simulink library "ee_lib_for_power_systems"  (R2025b) (continued)
== system ee_lib_for_power_systems/Network Components == (continued)
      | hC = find_system(gcbh,'FollowLinks','on','FindAll','on','IncludeCommented','on','LookUnderMasks','on','Name','Capacitor');
      | 
      | set(hR,'Commented','off')
      | set(hL,'Commented','off')
      | set(hC,'Commented','off')
      | 
      | if R == Inf
      |     set(hR,'Commented','on')
      | end
      | 
      | if L == Inf
      |     set(hL,'Commented','on')
      | end
      | 
      | if C == 0
      |     set(hC,'Commented','on')
      | end
      mask Callback run_time:
      | run_time = get_param(gcbh,'run_time');
      | 
      | hR = find_system(gcbh,'FollowLinks','on','FindAll','on','IncludeCommented','on','LookUnderMasks','on','Name','Resistor');
      | hL = find_system(gcbh,'FollowLinks','on','FindAll','on','IncludeCommented','on','LookUnderMasks','on','Name','Inductor');
      | hC = find_system(gcbh,'FollowLinks','on','FindAll','on','IncludeCommented','on','LookUnderMasks','on','Name','Capacitor');
      | 
      | switch run_time
      |     case 'off'
      |         pcr = 'compiletime';
      |     case 'on'
      |         pcr = 'runtime';
      | end
      | 
      | set(hR,'R_conf',pcr)
      | set(hL,'l_conf',pcr)
      | set(hC,'c_conf',pcr)
      mask DialogControl DescGroupVar Prompt: Parallel Z - RLC
      mask DialogControl DescTextVar Prompt: Define constant parallel impedance through R, L and C values. Set R = Inf to remove the resistor, set L = Inf to remove the inductor and set C = 0 to remove the capacitor.
      mask Display: disp('Parallel Z - RLC')
  Passive : ee_lib/Passive  Tag=simscape_sublibrary  SystemSampleTime=-1  VariantActivationTime=update diagram  EmptyChoice=off  PassThrough=off  PassThroughVariantControl=(default)  EmptyChoiceVariantControl=(default)  AllowFlexibleInterface=on  Latency=0  AutoFrameSizeCalculation=off
  Pi-Section Pos-Zero (PU) : Subsystem  mask{length=1, Pnom_Vnom_Fnom=[5e6 5e3 60], R1_L1_C1=[2 0.05 1e-8], R0_L0_C0=[4 0.1 1e-09], run_time=off}
      mask prompts: length "Line Length (km)"; Pnom_Vnom_Fnom "Nominal values [Pnom(VA) Vnom(Vrms) Fnom(Hz)]"; R1_L1_C1 "Positive Sequence [R1(pu) L1(pu) C1(pu)]"; R0_L0_C0 "Zero Sequence [R0(pu) L0(pu) C0(pu)]"; run_time "Set parameters to run-time (model does not recompile if parameters change value)"
      mask init:
      | maskObj = Simulink.Mask.get(gcbh);
      | workspaceObj = maskObj.getWorkspace();
      | 
      | Pnom_Vnom_Fnom = workspaceObj.get('Pnom_Vnom_Fnom');
      | R1_L1_C1 = workspaceObj.get('R1_L1_C1');
      | R0_L0_C0 = workspaceObj.get('R0_L0_C0');
      | 
      | Pnom = Pnom_Vnom_Fnom(1);
      | Vnom = Pnom_Vnom_Fnom(2);
      | Fnom = Pnom_Vnom_Fnom(3);
      | 
      | Rbase = Vnom^2/Pnom(1);
      | Lbase = Rbase/(2*pi*Fnom);
      | Cbase = 1/Rbase/(2*pi*Fnom);
      | 
      | R1_si = R1_L1_C1(1)*Rbase;
      | L1_si = R1_L1_C1(2)*Lbase;
      | C1_si = R1_L1_C1(3)*Cbase;
      | R0_si = R0_L0_C0(1)*Rbase;
      | L0_si = R0_L0_C0(2)*Lbase;
      | C0_si = R0_L0_C0(3)*Cbase;
      | 
      | Rs_si = (2*R1_si + R0_si)/3;
      | Rm_si = (R0_si - R1_si)/3;
      | 
      | Ls_si = (2*L1_si + L0_si)/3;
      | Lm_si = (L0_si - L1_si)/3;
      | 
      | Cl_si = (C1_si-C0_si)/3;
      | Cg_si = C0_si;
      mask Callback run_time:
      | run_time = get_param(gcbh,'run_time');
      | 
      | path_line = [gcb,'/line'];
      | 
      | switch run_time
      |     case 'off'
      |         pcr = 'compiletime';
      |     case 'on'
      |         pcr = 'runtime';
      | end
      | 
      | set_param(path_line,'freq_conf',pcr)
      | set_param(path_line,'length_conf',pcr)
      | set_param(path_line,'R_conf',pcr)
      | set_param(path_line,'L_conf',pcr)
      | set_param(path_line,'Rm_conf',pcr)
      | set_param(path_line,'M_conf',pcr)
      | set_param(path_line,'Cg_conf',pcr)
      | set_param(path_line,'Cl_conf',pcr)
      mask DialogControl DescGroupVar Prompt: Pi-Section Pos-Zero
      mask DialogControl DescTextVar Prompt: Define pi-section line with positive and zero sequence values
      mask DialogControl Container5 Prompt: Parameters
      mask Display: disp('Pi-Section\nPos-Zero\n(PU)')
  Pi-Section Pos-Zero (SI) : Subsystem  mask{length=1, Fnom=60, R1_L1_C1=[0.02 0.05 1e-8], R0_L0_C0=[0.04 4 0.1 1e-09], run_time=off}
      mask prompts: length "Line Length (km)"; Fnom "Nominal Frequency (Hz)"; R1_L1_C1 "Positive Sequence [R1(Ohm/km) L1(H/km) C1(F/km)]"; R0_L0_C0 "Zero Sequence [R0(Ohm/km) L0(H/km) C0(F/km)]"; run_time "Set parameters to run-time (model does not recompile if parameters change value)"
      mask init:
      | maskObj = Simulink.Mask.get(gcbh);
      | workspaceObj = maskObj.getWorkspace();
      | 
      | R1_L1_C1 = workspaceObj.get('R1_L1_C1');
      | R0_L0_C0 = workspaceObj.get('R0_L0_C0');
      | 
      | R1_si = R1_L1_C1(1);
      | L1_si = R1_L1_C1(2);
      | C1_si = R1_L1_C1(3);
      | R0_si = R0_L0_C0(1);
      | L0_si = R0_L0_C0(2);
      | C0_si = R0_L0_C0(3);
      | 
      | Rs_si = (2*R1_si + R0_si)/3;
      | Rm_si = (R0_si - R1_si)/3;
      | 
      | Ls_si = (2*L1_si + L0_si)/3;
      | Lm_si = (L0_si - L1_si)/3;
      | 
      | Cl_si = (C1_si-C0_si)/3;
      | Cg_si = C0_si;
      mask Callback run_time:
      | run_time = get_param(gcb,'run_time');
      | 
      | path_line = [gcb,'/line'];
      | 
      | switch run_time
      |     case 'off'
      |         pcr = 'compiletime';
      |     case 'on'
      |         pcr = 'runtime';
      | end
      | 
      | set_param(path_line,'freq_conf',pcr)
      | set_param(path_line,'length_conf',pcr)
      | set_param(path_line,'R_conf',pcr)
      | set_param(path_line,'L_conf',pcr)
      | set_param(path_line,'Rm_conf',pcr)
      | set_param(path_line,'M_conf',pcr)
      | set_param(path_line,'Cg_conf',pcr)
      | set_param(path_line,'Cl_conf',pcr)
      mask DialogControl DescGroupVar Prompt: Pi-Section Pos-Zero (SI)
      mask DialogControl DescTextVar Prompt: Define pi-section line with positive and zero sequence values
      mask DialogControl Container5 Prompt: Parameters
      mask Display: disp('Pi-Section\nPos-Zero\n(SI)')
  Resistor (Table-Based) : Subsystem  mask{i_vec=[1 2 3 4], v_vec=[1 2 3 4], run_time=off}
      mask prompts: i_vec "Current vector (A)"; v_vec "Voltage vector (V)"; run_time "Set parameters to run-time (model does not recompile if parameters change value)"
      mask Callback run_time:
      | h = find_system(gcbh,'FollowLinks','on','FindAll','on','IncludeCommented','on','LookUnderMasks','on','Name','LUT');
      | 
      | run_time = get(gcbh,'run_time');
      | 
      | switch run_time
      | 
      |     case 'on'
      | 
      |         set(h,'x_conf','runtime')
      |         set(h,'f_conf','runtime')
      | 
      |     case 'off'
      | 
      |         set(h,'x_conf','compiletime')
      |         set(h,'f_conf','compiletime')
      | 
      | end
      mask Display: disp('Resistor\n(Table-Based)')
  Series Z - PQ : Subsystem  mask{Vnom_Fnom=[100 60], P=1e3, Q=1e3, run_time=off}
      mask prompts: Vnom_Fnom "Nominal Values [Vnom(Vrms) Fnom(Hz)]"; P "Active Power (W)"; Q "Reactive Power  (inductive +VAr, capacitive -VAr )"; run_time "Set parameters to run-time (model does not recompile if parameters change value)"
      mask init:
      | maskObj = Simulink.Mask.get(gcbh);
      | workspaceObj = maskObj.getWorkspace();
      | 
      | Vnom_Fnom = workspaceObj.get('Vnom_Fnom');
      | P = workspaceObj.get('P');
      | Q = workspaceObj.get('Q');
      | 
      | path_R   = [gcb,'/Resistor'];
      | path_L   = [gcb,'/Inductor'];
      | path_C   = [gcb,'/Capacitor'];
      | 
      | Vnom = Vnom_Fnom(1);
      | Fnom = Vnom_Fnom(2);
      | 
      | set_param(path_R,'Commented','off')
      | set_param(path_L,'Commented','off')
      | set_param(path_C,'Commented','off')
      | 
      | S = sqrt(P^2 + Q^2);
      | I = S/Vnom;
      | R = P/(I^2);
      | C = 1e-6;
      | L = 1e-6;
      | 
      | if P == 0
      |     set_param(path_R,'Commented','through')
      | end
      | 
      | if Q > 0
      |     L = Q/(I^2)/(2*pi*Fnom);
      |     set_param(path_C,'Commented','through')
      | elseif Q < 0
      |     C = (I^2)/abs(Q)/(2*pi*Fnom);
      |     set_param(path_L,'Commented','through')
      | elseif Q == 0
      |     set_param(path_L,'Commented','through')
      |     set_param(path_C,'Commented','through')
      | end
      mask Callback run_time:
      | run_time = get_param(gcb,'run_time');
      | 
      | path_R   = [gcb,'/Resistor'];
      | path_L   = [gcb,'/Inductor'];
      | path_C   = [gcb,'/Capacitor'];
      | 
      | switch run_time
      |     case 'off'
      |         pcr = 'compiletime';
      |     case 'on'
      |         pcr = 'runtime';
      | end
      | 
      | set_param(path_R,'R_conf',pcr)
      | set_param(path_L,'l_conf',pcr)
      | set_param(path_C,'c_conf',pcr)
      mask DialogControl DescGroupVar Prompt: Series Z - PQ
      mask DialogControl DescTextVar Prompt: Define series constant impedance through P and Q values
      mask Display: disp('Series Z - PQ')
  Series Z - RLC : Subsystem  mask{R=0, L=0.001, C=1e-5, run_time=off}
      mask prompts: R "Resistance (Ohm)"; L "Inductance (H)"; C "Capacitance (F)"; run_time "Set parameters to run-time (model does not recompile if parameters change value)"
      mask init:
      | maskObj = Simulink.Mask.get(gcbh);
      | workspaceObj = maskObj.getWorkspace();
      | 
      | R = workspaceObj.get('R');
      | L = workspaceObj.get('L');
      | C = workspaceObj.get('C');
      | 
      | 
      | hR = find_system(gcbh,'FollowLinks','on','FindAll','on','IncludeCommented','on','LookUnderMasks','on','Name','Resistor');
      | hL = find_system(gcbh,'FollowLinks','on','FindAll','on','IncludeCommented','on','LookUnderMasks','on','Name','Inductor');
      | hC = find_system(gcbh,'FollowLinks','on','FindAll','on','IncludeCommented','on','LookUnderMasks','on','Name','Capacitor');
      | 
      | set(hR,'Commented','off')
      | set(hL,'Commented','off')
      | set(hC,'Commented','off')
      | 
      | if R == 0
      |     set(hR,'Commented','through')
      | end
      | 
      | if L == 0
      |     set(hL,'Commented','through')
      | end
      | 
      | if C == Inf
      |     set(hC,'Commented','through')
      | end
      mask Callback run_time:
      | run_time = get_param(gcbh,'run_time');
      | 
      | hR = find_system(gcbh,'FollowLinks','on','FindAll','on','IncludeCommented','on','LookUnderMasks','on','Name','Resistor');
      | hL = find_system(gcbh,'FollowLinks','on','FindAll','on','IncludeCommented','on','LookUnderMasks','on','Name','Inductor');
      | hC = find_system(gcbh,'FollowLinks','on','FindAll','on','IncludeCommented','on','LookUnderMasks','on','Name','Capacitor');
      | 
      | switch run_time
      |     case 'off'
      |         pcr = 'compiletime';
      |     case 'on'
      |         pcr = 'runtime';
      | end
      | 
      | set(hR,'R_conf',pcr)
      | set(hL,'l_conf',pcr)
      | set(hC,'c_conf',pcr)
      mask DialogControl DescGroupVar Prompt: Series Z - RLC
      mask DialogControl DescTextVar Prompt: Define constant series impedance through R, L and C values. Set R = 0 to remove the resistor, set L = 0 to remove the inductor and set C = Inf to remove the capacitor.
      mask Display: disp('Series Z - RLC')
  Simulink Logic Breaker : Subsystem  mask{initB=1, Ron=1e-6, Goff=1e-6, Ts=5e-5}
      mask prompts: initB "Initial Breaker Status (0 open, 1 closed)"; Ron "On Resistance (Ohm)"; Goff "Off Conductance (1/Ohm)"; Ts "Sample Time (sec)"
      settings: PortSchema="{\"entries\":[{\"content\":{\"sides\":[{\"content\":{\"connectorIds\":[\"LConn1\"]},\"type\":\"ConnectorPlacement.EquallySpacedRectSide\",\"uuid\":\"ba0bfa42-8952-44f0-a781-b329520855ba\"},{\"content\":{\"connectorIds\":[\"RConn1\"],\"side\":\"RIGHT\"},\"type\":\"ConnectorPlacement.EquallySpacedRectSide\",\"uuid\":\"e904d15c-eff7-47ac-9c24-60f529d3b54e\"},{\"content\":{\"connectorIds\":[\"In1\"],\"side\":\"TOP\"},\"type\":\"ConnectorPlacement.EquallySpacedRectSide\",\"uuid\":\"ccdae53b-1241-4ab5-a4dd-bd861eec6f9e\"}]},\"type\":\"ConnectorPlacement.EquallySpacedConnector\",\"uuid\":\"4cc3036f-f6ff-4340-9c1c-9c60c081c1a2\"}],\"packageUris\":[\"http://schema.mathworks.com/mf0/Connector/R2020b\"],\"version\":\"1.0\"}"
      mask DialogControl DescGroupVar Prompt: Single-Phase Breaker
      mask DialogControl DescTextVar Prompt: Breaker is closed when signal is 1. Breaker opens when signal goes to zero and current crosses zero.
      mask Display: disp('Breaker')
  Simulink Logic Breaker (Three-Phase) : Subsystem  mask{initB=1, Ron=1e-6, Goff=1e-6, Ts=5e-5}
      mask prompts: initB "Initial Breaker Status (0 open, 1 closed)"; Ron "On Resistance (Ohm)"; Goff "Off Conductance (1/Ohm)"; Ts "Sample Time (sec)"
      settings: PortSchema="{\"entries\":[{\"content\":{\"sides\":[{\"content\":{\"connectorIds\":[\"LConn1\"]},\"type\":\"ConnectorPlacement.EquallySpacedRectSide\",\"uuid\":\"ba0bfa42-8952-44f0-a781-b329520855ba\"},{\"content\":{\"connectorIds\":[\"RConn1\"],\"side\":\"RIGHT\"},\"type\":\"ConnectorPlacement.EquallySpacedRectSide\",\"uuid\":\"e904d15c-eff7-47ac-9c24-60f529d3b54e\"},{\"content\":{\"connectorIds\":[\"In1\"],\"side\":\"TOP\"},\"type\":\"ConnectorPlacement.EquallySpacedRectSide\",\"uuid\":\"ccdae53b-1241-4ab5-a4dd-bd861eec6f9e\"}]},\"type\":\"ConnectorPlacement.EquallySpacedConnector\",\"uuid\":\"4cc3036f-f6ff-4340-9c1c-9c60c081c1a2\"}],\"packageUris\":[\"http://schema.mathworks.com/mf0/Connector/R2020b\"],\"version\":\"1.0\"}"
      mask DialogControl DescGroupVar Prompt: Three-Phase Breaker
      mask DialogControl DescTextVar Prompt: Breaker is closed when signal is 1. Breaker opens when signal goes to zero and current crosses zero.
      mask Display: disp('Breaker')
  Switches & Breakers : ee_lib/Switches & Breakers  Tag=simscape_sublibrary  SystemSampleTime=-1  VariantActivationTime=update diagram  EmptyChoice=off  PassThrough=off  PassThroughVariantControl=(default)  EmptyChoiceVariantControl=(default)  AllowFlexibleInterface=on  Latency=0  AutoFrameSizeCalculation=off
  Transformers : ee_lib/Passive/Transformers  Tag=simscape_sublibrary  SystemSampleTime=-1  VariantActivationTime=update diagram  EmptyChoice=off  PassThrough=off  PassThroughVariantControl=(default)  EmptyChoiceVariantControl=(default)  AllowFlexibleInterface=on  Latency=0  AutoFrameSizeCalculation=off
  Two-Winding Transformer Nameplate (Three-Phase) : Subsystem  mask{conn1=Y, conn2=Y, core=threelimb, Prated=100, Frated=60, HV_LV=[11000 415], HVA_LVA=[5.25 139.1], Zperc=3.88, load_loss=1750, no_load_loss=145, run_time=off}
      mask prompts: conn1 "Winding 1 Connection"; conn2 "Winding 2 Connection"; Prated "Rated Power (kVA)"; Frated "Rated Frequency (Hz)"; HV_LV "Rated Voltage (Vrms line-line) [HV LV]"; HVA_LVA "Rated Current (A line peak) [HV Amps LV Amps]"; Zperc "Short-Circuit Impedance (%)"; load_loss "Copper (Load) Loss (W)"; no_load_loss "Core (No-Load) Loss (W)"; run_time "Set parameters to run-time (model does not recompile if parameters change value)"
      mask init:
      | maskObj = Simulink.Mask.get(gcbh);
      | workspaceObj = maskObj.getWorkspace();
      | 
      | Prated = workspaceObj.get('Prated');
      | Frated = workspaceObj.get('Frated');
      | HV_LV = workspaceObj.get('HV_LV');
      | HVA_LVA = workspaceObj.get('HVA_LVA');
      | Zperc = workspaceObj.get('Zperc');
      | load_loss = workspaceObj.get('load_loss');
      | no_load_loss = workspaceObj.get('no_load_loss');
      | 
      | HV = HV_LV(1);
      | LV = HV_LV(2);
      | HVA = HVA_LVA(1);
      | LVA = HVA_LVA(2);
      | 
      | P_rated_peak_per_phase = sqrt(2)*Prated/3*1000;
      | V_rated_peak_phase_to_ground = HV*sqrt(2/3);
      | 
      | Rbase1_per_phase = V_rated_peak_phase_to_ground^2/P_rated_peak_per_phase;
      | 
      | Rm = V_rated_peak_phase_to_ground^2/(sqrt(2)*no_load_loss/3);
      | 
      | Rm_pu = Rm/Rbase1_per_phase;
      | 
      | R1_pu = (sqrt(2)*load_loss/3)/(HVA^2)/Rbase1_per_phase/2;
      | R2_pu = R1_pu;
      | 
      | L1_pu = sqrt((sqrt(1/2)*Zperc/100)^2-R1_pu^2);
      | L2_pu = L1_pu;
      | 
      | path_Transformer = [gcb,'/Transformer'];
      | 
      | conn1 = get_param(gcb,'conn1');
      | conn2 = get_param(gcb,'conn2');
      | core  = get_param(gcb,'core');
      | 
      | set_param(path_Transformer,'Winding1Connection',['ee.enum.windingconnection.',conn1])
      | set_param(path_Transformer,'Winding2Connection',['ee.enum.windingconnection.',conn2])
      | set_param(path_Transformer,'CoreType',['ee.enum.coretype.',core])
      mask TypeOptions conn1: Y | Yg | delta1 | delta11
      mask TypeOptions conn2: Y | Yg | delta1 | delta11
      mask TypeOptions core: threelimb | fivelimb
      mask DialogControl DescGroupVar Prompt: Two-Winding Transformer (Nameplate)
      mask DialogControl Container4 Prompt: Configure
      mask DialogControl Container5 Prompt: Nameplate Data
      mask Display:
      | color('lightblue')
      | 
      | ph = linspace(0,2*pi,200);
      | 
      | plot(0.15*sin(ph)+0.4,0.15*cos(ph)+0.5)
      | plot(0.15*sin(ph)+0.6,0.15*cos(ph)+0.5)
      | plot([0.2 0.25],[0.5 0.5])
      | plot([0.75 0.8],[0.5 0.5])
      | plot(0,0)
      | plot(1,1)
      | 
      | conn1 = get_param(gcbh,'conn1');
      | cc1 = conn1;
      | if strcmp(conn1,'delta1')
      |     cc1 = 'D1';
      | elseif strcmp(conn1,'delta11')
      |     cc1 = 'D11';
      | end
      | 
      | text(0.2,0.25,cc1)
      | 
      | conn2 = get_param(gcbh,'conn2');
      | cc2 = conn2;
      | if strcmp(conn2,'delta1')
      |     cc2 = 'D1';
      | elseif strcmp(conn2,'delta11')
      |     cc2 = 'D11';
      | end
      | 
      | text(0.7,0.25,cc2)
      | 
      | core = get_param(gcbh,'core');
      | if strcmp(core,'threelimb')
      |     co1 = '3-Limb';
      | elseif strcmp(core,'fivelimb')
      |     co1 = '5-Limb';
      | end
      | 
      | text(0.32,0.8,co1)
  Two-Winding Transformer PU (Three-Phase) : Subsystem  mask{conn1=Yg, conn2=delta1, core=threelimb, Pnom_Fnom=[250e6 60], V1_V2=[500000 250000], R_X=[0.01 0.05], Rm_Xm=[500 500], run_time=off}
      mask prompts: conn1 "Winding 1 Connection"; conn2 "Winding 2 Connection"; Pnom_Fnom "Nominal values [Pnom(VA) Fnom(Hz)]"; V1_V2 "Primary and Secondary Voltages[V1(Vrms) V2(Vrms)]"; R_X "Winding Resistance and Leakage Reactance [R(pu) X(pu)]"; Rm_Xm "Magnetization [Rm(pu) Xm(pu)]"; run_time "Set parameters to run-time (model does not recompile if parameters change value)"
      mask init:
      | maskObj = Simulink.Mask.get(gcbh);
      | workspaceObj = maskObj.getWorkspace();
      | 
      | Pnom_Fnom = workspaceObj.get('Pnom_Fnom');
      | V1_V2 = workspaceObj.get('V1_V2');
      | R_X = workspaceObj.get('R_X');
      | Rm_Xm = workspaceObj.get('Rm_Xm');
      | 
      | V1 = V1_V2(1);
      | V2 = V1_V2(2);
      | 
      | R1_pu = R_X(1)/2;
      | L1_pu = R_X(2)/2;
      | R2_pu = R_X(1)/2;
      | L2_pu = R_X(2)/2;
      | Rm_pu = Rm_Xm(1);
      | Lm_pu = Rm_Xm(2);
      | 
      | conn1 = get_param(gcb,'conn1');
      | conn2 = get_param(gcb,'conn2');
      | core  = get_param(gcb,'core');
      | 
      | 
      | path_Transformer = [gcb,'/Transformer'];
      | 
      | set_param(path_Transformer,'Winding1Connection',['ee.enum.windingconnection.',conn1])
      | set_param(path_Transformer,'Winding2Connection',['ee.enum.windingconnection.',conn2])
      | set_param(path_Transformer,'CoreType',['ee.enum.coretype.',core])
      mask TypeOptions conn1: Y | Yg | delta1 | delta11
      mask TypeOptions conn2: Y | Yg | delta1 | delta11
      mask TypeOptions core: threelimb | fivelimb
      mask Callback run_time:
      | run_time = get_param(gcb,'run_time');
      | 
      | path_Transformer = [gcb,'/Transformer'];
      | 
      | switch run_time
      |     case 'off'
      |         pcr = 'compiletime';
      |     case 'on'
      |         pcr = 'runtime';
      | end
      | 
      | set_param(path_Transformer,'VRated1_conf',pcr)
      | set_param(path_Transformer,'VRated2_conf',pcr)
      | set_param(path_Transformer,'SRated_conf',pcr)
      | set_param(path_Transformer,'FRated_conf',pcr)
      | set_param(path_Transformer,'pu_Rm_conf',pcr)
      | set_param(path_Transformer,'pu_Xm_conf',pcr)
      | set_param(path_Transformer,'pu_Rw1_conf',pcr)
      | set_param(path_Transformer,'pu_Xl1_conf',pcr)
      | set_param(path_Transformer,'pu_Rw2_conf',pcr)
      | set_param(path_Transformer,'pu_Xl2_conf',pcr)
      mask DialogControl DescGroupVar Prompt: Two-Winding Transformer
      mask DialogControl DescTextVar Prompt: Winding resistance and leakage reactance are split equally between primary and secondary windings.
      mask DialogControl Container4 Prompt: Configure
      mask DialogControl Container5 Prompt: Parameters
      mask Display:
      | color('lightblue')
      | 
      | ph = linspace(0,2*pi,200);
      | 
      | plot(0.2*sin(ph)+0.4,0.2*cos(ph)+0.5)
      | plot(0.2*sin(ph)+0.6,0.2*cos(ph)+0.5)
      | plot([0.1 0.2],[0.5 0.5])
      | plot([0.8 0.9],[0.5 0.5])
      | plot(0,0)
      | plot(1,1)
      | 
      | conn1 = get_param(gcbh,'conn1');
      | cc1 = conn1;
      | if strcmp(conn1,'delta1')
      |     cc1 = 'D1';
      | elseif strcmp(conn1,'delta11')
      |     cc1 = 'D11';
      | end
      | 
      | text(0.2,0.25,cc1)
      | 
      | conn2 = get_param(gcbh,'conn2');
      | cc2 = conn2;
      | if strcmp(conn2,'delta1')
      |     cc2 = 'D1';
      | elseif strcmp(conn2,'delta11')
      |     cc2 = 'D11';
      | end
      | 
      | text(0.7,0.25,cc2)
      | 
      | core = get_param(gcbh,'core');
      | if strcmp(core,'threelimb')
      |     co1 = '3-Limb';
      | elseif strcmp(core,'fivelimb')
      |     co1 = '5-Limb';
      | end
      | 
      | text(0.32,0.8,co1)
  Variable C : Subsystem  mask{Cmin=1e-9, Rseries=1e-6}
      mask prompts: Cmin "Minimum Capacitance (F)"; Rseries "Series Resistance (Ohm)"
      settings: PortSchema="{\"entries\":[{\"content\":{\"sides\":[{\"content\":{\"connectorIds\":[\"LConn1\"]},\"type\":\"ConnectorPlacement.EquallySpacedRectSide\",\"uuid\":\"090b2ee3-4378-4784-ad56-1af8514f6806\"},{\"content\":{\"connectorIds\":[\"RConn1\"],\"side\":\"RIGHT\"},\"type\":\"ConnectorPlacement.EquallySpacedRectSide\",\"uuid\":\"4adbd240-d61e-4082-b4ef-02f0dbd361b1\"},{\"content\":{\"connectorIds\":[\"In1\"],\"side\":\"TOP\"},\"type\":\"ConnectorPlacement.EquallySpacedRectSide\",\"uuid\":\"34d099db-9580-46ff-aa35-d8c98d9402ef\"}]},\"type\":\"ConnectorPlacement.EquallySpacedConnector\",\"uuid\":\"cb3dc6bb-ab47-4bf2-a22f-b68fe84c97d5\"}],\"packageUris\":[\"http://schema.mathworks.com/mf0/Connector/R2020b\"],\"version\":\"1.0\"}"
      mask Display: disp('Variable C')
  Variable L : Subsystem  mask{Lmin=1e-9, Gpar=1e-6}
      mask prompts: Lmin "Minimum Inductance (H)"; Gpar "Parallel Conductance (1/Ohm)"
      settings: PortSchema="{\"entries\":[{\"content\":{\"sides\":[{\"content\":{\"connectorIds\":[\"LConn1\"]},\"type\":\"ConnectorPlacement.EquallySpacedRectSide\",\"uuid\":\"090b2ee3-4378-4784-ad56-1af8514f6806\"},{\"content\":{\"connectorIds\":[\"RConn1\"],\"side\":\"RIGHT\"},\"type\":\"ConnectorPlacement.EquallySpacedRectSide\",\"uuid\":\"4adbd240-d61e-4082-b4ef-02f0dbd361b1\"},{\"content\":{\"connectorIds\":[\"In1\"],\"side\":\"TOP\"},\"type\":\"ConnectorPlacement.EquallySpacedRectSide\",\"uuid\":\"34d099db-9580-46ff-aa35-d8c98d9402ef\"}]},\"type\":\"ConnectorPlacement.EquallySpacedConnector\",\"uuid\":\"cb3dc6bb-ab47-4bf2-a22f-b68fe84c97d5\"}],\"packageUris\":[\"http://schema.mathworks.com/mf0/Connector/R2020b\"],\"version\":\"1.0\"}"
      mask Display: disp('Variable L')
  Variable R : Subsystem  mask{Rmin=1e-6}
      mask prompts: Rmin "Minimum Resistance (Ohm)"
      settings: PortSchema="{\"entries\":[{\"content\":{\"sides\":[{\"content\":{\"connectorIds\":[\"LConn1\"]},\"type\":\"ConnectorPlacement.EquallySpacedRectSide\",\"uuid\":\"090b2ee3-4378-4784-ad56-1af8514f6806\"},{\"content\":{\"connectorIds\":[\"RConn1\"],\"side\":\"RIGHT\"},\"type\":\"ConnectorPlacement.EquallySpacedRectSide\",\"uuid\":\"4adbd240-d61e-4082-b4ef-02f0dbd361b1\"},{\"content\":{\"connectorIds\":[\"In1\"],\"side\":\"TOP\"},\"type\":\"ConnectorPlacement.EquallySpacedRectSide\",\"uuid\":\"34d099db-9580-46ff-aa35-d8c98d9402ef\"}]},\"type\":\"ConnectorPlacement.EquallySpacedConnector\",\"uuid\":\"cb3dc6bb-ab47-4bf2-a22f-b68fe84c97d5\"}],\"packageUris\":[\"http://schema.mathworks.com/mf0/Connector/R2020b\"],\"version\":\"1.0\"}"
      mask Display: disp('Variable R')
  Variable Transformer : Subsystem
      settings: PortSchema="{\"entries\":[{\"content\":{\"sides\":[{\"content\":{\"connectorIds\":[\"LConn1\",\"LConn2\"]},\"type\":\"ConnectorPlacement.EquallySpacedRectSide\",\"uuid\":\"e266206b-9189-4395-81ab-f92d066fdce2\"},{\"content\":{\"connectorIds\":[\"RConn1\",\"RConn2\"],\"side\":\"RIGHT\"},\"type\":\"ConnectorPlacement.EquallySpacedRectSide\",\"uuid\":\"f4c76213-e35b-4eb7-9178-e126ecdfb00b\"},{\"content\":{\"connectorIds\":[\"In1\"],\"side\":\"TOP\"},\"type\":\"ConnectorPlacement.EquallySpacedRectSide\",\"uuid\":\"d98ac605-465f-4e96-bdfd-c241a94d6f41\"}]},\"type\":\"ConnectorPlacement.EquallySpacedConnector\",\"uuid\":\"ee260325-4efb-4256-abf4-45aef0fdc09a\"}],\"packageUris\":[\"http://schema.mathworks.com/mf0/Connector/R2020b\"],\"version\":\"1.0\"}"
      mask DialogControl Container1 Prompt: Variable transfomer
      mask DialogControl Control1 Prompt: V2 = N*V1 and I1 = -N*V2
      mask Display:
      | color('blue')
      | 
      | phl = linspace(0,-pi,200);
      | phr = linspace(0,pi,200);
      | 
      | r1 = 0.07;
      | b1 = 0.3;
      | 
      | plot(r1*sin(phl)+0.44,r1*cos(phl)+b1)
      | plot(r1*sin(phl)+0.44,r1*cos(phl)+b1+2*r1)
      | plot(r1*sin(phl)+0.44,r1*cos(phl)+b1+4*r1)
      | plot(r1*sin(phl)+0.44,r1*cos(phl)+b1+6*r1)
      | 
      | plot(r1*sin(phr)+0.56,r1*cos(phr)+b1)
      | plot(r1*sin(phr)+0.56,r1*cos(phr)+b1+2*r1)
      | plot(r1*sin(phr)+0.56,r1*cos(phr)+b1+4*r1)
      | plot(r1*sin(phr)+0.56,r1*cos(phr)+b1+6*r1)
      | 
      | 
      | %plot(0.05*sin(phr)+0.6,0.05*cos(phr)+0.5)
      | 
      | plot(0,0)
      | plot(1,1)
      | plot([0.2 0.44],[0.85 0.85])
      | plot([0.56 0.8],[0.85 0.85])
      | plot([0.2 0.44],[0.15 0.15])
      | plot([0.56 0.8],[0.15 0.15])
      | plot([0.56 0.56],[0.85 0.79])
      | plot([0.44 0.44],[0.85 0.79])
      | plot([0.56 0.56],[0.15 0.23])
      | plot([0.44 0.44],[0.15 0.23])
  Zigzag-Delta Transformer PU (Three-Phase) : Subsystem  mask{conn2=delta11, Pnom_Fnom=[250e6 60], V1_V2=[500000 250000], R_X=[0.01 0.05], Rm_Xm=[500 500], phase=-7.5, run_time=off}
      mask prompts: conn2 "Delta Connection"; Pnom_Fnom "Nominal values [Pnom(VA) Fnom(Hz)]"; V1_V2 "Primary and Secondary Voltages[V1(Vrms) V2(Vrms)]"; R_X "Winding Resistance and Leakage Reactance [R(pu) X(pu)]"; Rm_Xm "Magnetization [Rm(pu) Xm(pu)]"; phase "Secondary Phase Shift (deg)"; run_time "Set parameters to run-time (model does not recompile if parameters change value)"
      mask init:
      | maskObj = Simulink.Mask.get(gcbh);
      | workspaceObj = maskObj.getWorkspace();
      | 
      | Pnom_Fnom = workspaceObj.get('Pnom_Fnom');
      | V1_V2 = workspaceObj.get('V1_V2');
      | R_X = workspaceObj.get('R_X');
      | Rm_Xm = workspaceObj.get('Rm_Xm');
      | 
      | V1 = V1_V2(1);
      | V2 = V1_V2(2);
      | 
      | R1_pu = R_X(1)/2;
      | L1_pu = R_X(2)/2;
      | R2_pu = R_X(1)/2;
      | L2_pu = R_X(2)/2;
      | Rm_pu = Rm_Xm(1);
      | Lm_pu = Rm_Xm(2);
      | 
      | conn2 = get_param(gcb,'conn2');
      | 
      | path_Transformer = [gcb,'/Transformer'];
      | 
      | set_param(path_Transformer,'deltaconnection_option',['ee.enum.deltaconnection.',conn2])
      mask TypeOptions conn2: delta1 | delta11
      mask Callback run_time:
      | run_time = get_param(gcb,'run_time');
      | 
      | path_Transformer = [gcb,'/Transformer'];
      | 
      | switch run_time
      |     case 'off'
      |         pcr = 'compiletime';
      |     case 'on'
      |         pcr = 'runtime';
      | end
      | 
      | set_param(path_Transformer,'VRated1_conf',pcr)
      | set_param(path_Transformer,'VRated2_conf',pcr)
      | set_param(path_Transformer,'SRated_conf',pcr)
      | set_param(path_Transformer,'FRated_conf',pcr)
      | set_param(path_Transformer,'pu_Rm_conf',pcr)
      | set_param(path_Transformer,'pu_Xm_conf',pcr)
      | set_param(path_Transformer,'pu_Rw1_conf',pcr)
      | set_param(path_Transformer,'pu_Xl1_conf',pcr)
      | set_param(path_Transformer,'pu_Rw2_conf',pcr)
      | set_param(path_Transformer,'pu_Xl2_conf',pcr)
      | set_param(path_Transformer,'phaseShift_conf',pcr)
      mask DialogControl DescGroupVar Prompt: Zigzag-Delta Transformer
      mask DialogControl DescTextVar Prompt: Winding resistance and leakage reactance are split equally between primary and secondary windings.
      mask DialogControl Container4 Prompt: Configure
      mask DialogControl Container5 Prompt: Parameters
      mask Display:
      | color('lightblue')
      | 
      | ph = linspace(0,2*pi,200);
      | 
      | plot([0.1 0.2],[0.5 0.5])
      | plot([0.8 0.9],[0.5 0.5])
      | plot(0,0)
      | plot(1,1)
      | 
      | conn2 = get_param(gcbh,'conn2');
      | cc2 = conn2;
      | if strcmp(conn2,'delta1')
      |     cc2 = 'D1';
      | elseif strcmp(conn2,'delta11')
      |     cc2 = 'D11';
      | end
      | 
      | text(0.55,0.5,cc2)
      | text(0.3,0.5,'ZZ')
  multi-phase coupled RL : SimscapeComponentBlock  SourceFile=multi_coupled_RL  ComponentPath=multi_coupled_RL  ComponentVariants="[\"multi_coupled_RL\"]"  ComponentVariantNames="[\"multi_coupled_RL\"]"  ClassName=multi_coupled_RL  SchemaVersion=1  noph=3  R=[1 0.3 0.5;0.3 1 0.2;0.5 0.2 1] Ohm  L=[1 0.3 0.5;0.3 1 0.2;0.5 0.2 1]*1e-3 H
      settings: CopyFcn=simscape.sscblock.internal.componentBlockCallback(gcbh,'COPY')  PreCopyFcn=simscape.sscblock.internal.componentBlockCallback(gcbh,'PRECOPY')  PreDeleteFcn=simscape.sscblock.internal.componentBlockCallback(gcbh,'PREDELETE')  InternalSimscapePortConfiguration="{\"Bottom\":[],\"Left\":[{\"id\":\"p\",\"label\":\"p\",\"type\":\"foundation.electrical.electrical\"}],\"Right\":[{\"id\":\"n\",\"label\":\"n\",\"type\":\"foundation.electrical.electrical\"}],\"Top\":[]}"
      mask Type:
      | multi-phase
      | coupled RL
  multiphase DPL : SimscapeComponentBlock  SourceFile=mp_distributed_parameter_line  ComponentPath=mp_distributed_parameter_line  ComponentVariants="[\"mp_distributed_parameter_line\"]"  ComponentVariantNames="[\"mp_distributed_parameter_line\"]"  ClassName=mp_distributed_parameter_line  SchemaVersion=1  line_type=lineType.general  length=length km  freq=60 Hz  nPhase=3  N=1  Rs=0.02 Ohm/km  Rm=0.01 Ohm/km  Ls=0.5 mH/km  Lm=0.1 mH/km  Cs=0.7 uF/km  Cm=-0.3 uF/km  Rmat=R3m Ohm/km  Lmat=L3m*1e3 mH/km  Cmat=C3m*1e6 uF/km
      settings: CopyFcn=simscape.sscblock.internal.componentBlockCallback(gcbh,'COPY')  PreCopyFcn=simscape.sscblock.internal.componentBlockCallback(gcbh,'PRECOPY')  PreDeleteFcn=simscape.sscblock.internal.componentBlockCallback(gcbh,'PREDELETE')  InternalSimscapePortConfiguration="{\"Bottom\":[],\"Left\":[{\"id\":\"p\",\"label\":\"1\",\"type\":\"foundation.electrical.electrical\"}],\"Right\":[{\"id\":\"n\",\"label\":\"2\",\"type\":\"foundation.electrical.electrical\"}],\"Top\":[]}"
      mask Type: multiphase DPL
